simulink model "pfb_fft_vacc_float_fft"  (R2016b)
settings: Solver=VariableStepAuto  StartTime=0.0  StopTime=1024*2^5  RelTol=1e-3
undefined symbols (not defined in the file): fft_stages
libraries: xbsIndex_r4 x17526, casper_library_misc x189, casper_library_delays x15, casper_library_sources x4, xbsReplacements_r4 x3, xps_library x1
(part 30 of 140)

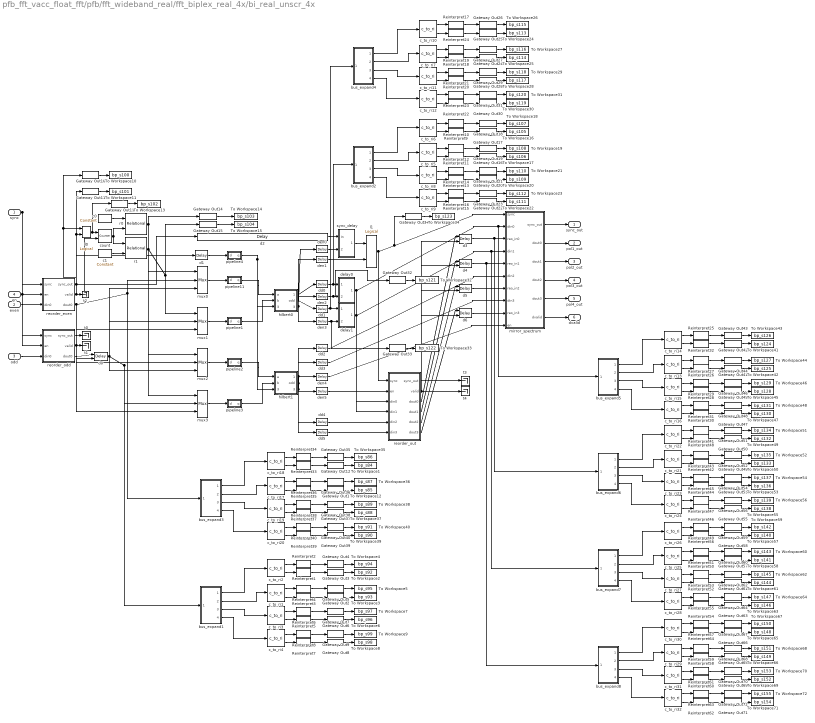
== system pfb_fft_vacc_float_fft/pfb/fft_wideband_real/fft_biplex_real_4x/bi_real_unscr_4x ==
  sync : Inport
  even : Inport  Port=2
  odd : Inport  Port=3
  en : Inport  Port=4
  Gateway Out{1..72} (72 alike) : xbsIndex_r4/Gateway Out  inherit_from_input=off  hdl_port=on  rows=1  columns=1  UseAsDAC=off  DACChannel='1'  interface=None  automatic_assignment=on  address_offset=hex2dec('00')  intf_name=''  intf_description=''  timing_constraint=None  locs_specified=off  LOCs={}  io_standard={}  block_type=gatewayout
  Reinterpret{1..64} (64 alike) : xbsIndex_r4/Reinterpret  force_arith_type=on  arith_type=Floating-point  force_bin_pt=on  bin_pt=24  block_type=reinterpret
  To Workspace1 : ToWorkspace  VariableName=bp_s84  SaveFormat=Timeseries  SampleTime=-1
  To Workspace10 : ToWorkspace  VariableName=bp_s100  SaveFormat=Timeseries  SampleTime=-1
  To Workspace11 : ToWorkspace  VariableName=bp_s101  SaveFormat=Timeseries  SampleTime=-1
  To Workspace12 : ToWorkspace  VariableName=bp_s85  SaveFormat=Timeseries  SampleTime=-1
  To Workspace13 : ToWorkspace  VariableName=bp_s102  SaveFormat=Timeseries  SampleTime=-1
  To Workspace14 : ToWorkspace  VariableName=bp_s103  SaveFormat=Timeseries  SampleTime=-1
  To Workspace15 : ToWorkspace  VariableName=bp_s104  SaveFormat=Timeseries  SampleTime=-1
  To Workspace16 : ToWorkspace  VariableName=bp_s105  SaveFormat=Timeseries  SampleTime=-1
  To Workspace17 : ToWorkspace  VariableName=bp_s106  SaveFormat=Timeseries  SampleTime=-1
  To Workspace18 : ToWorkspace  VariableName=bp_s107  SaveFormat=Timeseries  SampleTime=-1
  To Workspace19 : ToWorkspace  VariableName=bp_s108  SaveFormat=Timeseries  SampleTime=-1
  To Workspace2 : ToWorkspace  VariableName=bp_s92  SaveFormat=Timeseries  SampleTime=-1
  To Workspace20 : ToWorkspace  VariableName=bp_s109  SaveFormat=Timeseries  SampleTime=-1
  To Workspace21 : ToWorkspace  VariableName=bp_s110  SaveFormat=Timeseries  SampleTime=-1
  To Workspace22 : ToWorkspace  VariableName=bp_s111  SaveFormat=Timeseries  SampleTime=-1
  To Workspace23 : ToWorkspace  VariableName=bp_s112  SaveFormat=Timeseries  SampleTime=-1
  To Workspace24 : ToWorkspace  VariableName=bp_s113  SaveFormat=Timeseries  SampleTime=-1
  To Workspace25 : ToWorkspace  VariableName=bp_s114  SaveFormat=Timeseries  SampleTime=-1
  To Workspace26 : ToWorkspace  VariableName=bp_s115  SaveFormat=Timeseries  SampleTime=-1
  To Workspace27 : ToWorkspace  VariableName=bp_s116  SaveFormat=Timeseries  SampleTime=-1
  To Workspace28 : ToWorkspace  VariableName=bp_s117  SaveFormat=Timeseries  SampleTime=-1
  To Workspace29 : ToWorkspace  VariableName=bp_s118  SaveFormat=Timeseries  SampleTime=-1
  To Workspace3 : ToWorkspace  VariableName=bp_s93  SaveFormat=Timeseries  SampleTime=-1
  To Workspace30 : ToWorkspace  VariableName=bp_s119  SaveFormat=Timeseries  SampleTime=-1
  To Workspace31 : ToWorkspace  VariableName=bp_s120  SaveFormat=Timeseries  SampleTime=-1
  To Workspace32 : ToWorkspace  VariableName=bp_s121  SaveFormat=Timeseries  SampleTime=-1
  To Workspace33 : ToWorkspace  VariableName=bp_s122  SaveFormat=Timeseries  SampleTime=-1
  To Workspace34 : ToWorkspace  VariableName=bp_s123  SaveFormat=Timeseries  SampleTime=-1
  To Workspace35 : ToWorkspace  VariableName=bp_s86  SaveFormat=Timeseries  SampleTime=-1
  To Workspace36 : ToWorkspace  VariableName=bp_s87  SaveFormat=Timeseries  SampleTime=-1
  To Workspace37 : ToWorkspace  VariableName=bp_s88  SaveFormat=Timeseries  SampleTime=-1
  To Workspace38 : ToWorkspace  VariableName=bp_s89  SaveFormat=Timeseries  SampleTime=-1
  To Workspace39 : ToWorkspace  VariableName=bp_s90  SaveFormat=Timeseries  SampleTime=-1
  To Workspace4 : ToWorkspace  VariableName=bp_s94  SaveFormat=Timeseries  SampleTime=-1
  To Workspace40 : ToWorkspace  VariableName=bp_s91  SaveFormat=Timeseries  SampleTime=-1
  To Workspace41 : ToWorkspace  VariableName=bp_s124  SaveFormat=Timeseries  SampleTime=-1
  To Workspace42 : ToWorkspace  VariableName=bp_s125  SaveFormat=Timeseries  SampleTime=-1
  To Workspace43 : ToWorkspace  VariableName=bp_s126  SaveFormat=Timeseries  SampleTime=-1
  To Workspace44 : ToWorkspace  VariableName=bp_s127  SaveFormat=Timeseries  SampleTime=-1
  To Workspace45 : ToWorkspace  VariableName=bp_s128  SaveFormat=Timeseries  SampleTime=-1
  To Workspace46 : ToWorkspace  VariableName=bp_s129  SaveFormat=Timeseries  SampleTime=-1
  To Workspace47 : ToWorkspace  VariableName=bp_s130  SaveFormat=Timeseries  SampleTime=-1
  To Workspace48 : ToWorkspace  VariableName=bp_s131  SaveFormat=Timeseries  SampleTime=-1
  To Workspace49 : ToWorkspace  VariableName=bp_s132  SaveFormat=Timeseries  SampleTime=-1
  To Workspace5 : ToWorkspace  VariableName=bp_s95  SaveFormat=Timeseries  SampleTime=-1
  To Workspace50 : ToWorkspace  VariableName=bp_s133  SaveFormat=Timeseries  SampleTime=-1
  To Workspace51 : ToWorkspace  VariableName=bp_s134  SaveFormat=Timeseries  SampleTime=-1
  To Workspace52 : ToWorkspace  VariableName=bp_s135  SaveFormat=Timeseries  SampleTime=-1
  To Workspace53 : ToWorkspace  VariableName=bp_s136  SaveFormat=Timeseries  SampleTime=-1
  To Workspace54 : ToWorkspace  VariableName=bp_s137  SaveFormat=Timeseries  SampleTime=-1
  To Workspace55 : ToWorkspace  VariableName=bp_s138  SaveFormat=Timeseries  SampleTime=-1
  To Workspace56 : ToWorkspace  VariableName=bp_s139  SaveFormat=Timeseries  SampleTime=-1
  To Workspace57 : ToWorkspace  VariableName=bp_s140  SaveFormat=Timeseries  SampleTime=-1
  To Workspace58 : ToWorkspace  VariableName=bp_s141  SaveFormat=Timeseries  SampleTime=-1
  To Workspace59 : ToWorkspace  VariableName=bp_s142  SaveFormat=Timeseries  SampleTime=-1
  To Workspace6 : ToWorkspace  VariableName=bp_s96  SaveFormat=Timeseries  SampleTime=-1
  To Workspace60 : ToWorkspace  VariableName=bp_s143  SaveFormat=Timeseries  SampleTime=-1
  To Workspace61 : ToWorkspace  VariableName=bp_s144  SaveFormat=Timeseries  SampleTime=-1
  To Workspace62 : ToWorkspace  VariableName=bp_s145  SaveFormat=Timeseries  SampleTime=-1
  To Workspace63 : ToWorkspace  VariableName=bp_s146  SaveFormat=Timeseries  SampleTime=-1
  To Workspace64 : ToWorkspace  VariableName=bp_s147  SaveFormat=Timeseries  SampleTime=-1
  To Workspace65 : ToWorkspace  VariableName=bp_s148  SaveFormat=Timeseries  SampleTime=-1
  To Workspace66 : ToWorkspace  VariableName=bp_s149  SaveFormat=Timeseries  SampleTime=-1
  To Workspace67 : ToWorkspace  VariableName=bp_s150  SaveFormat=Timeseries  SampleTime=-1
  To Workspace68 : ToWorkspace  VariableName=bp_s151  SaveFormat=Timeseries  SampleTime=-1
  To Workspace69 : ToWorkspace  VariableName=bp_s152  SaveFormat=Timeseries  SampleTime=-1
  To Workspace7 : ToWorkspace  VariableName=bp_s97  SaveFormat=Timeseries  SampleTime=-1
  To Workspace70 : ToWorkspace  VariableName=bp_s153  SaveFormat=Timeseries  SampleTime=-1
  To Workspace71 : ToWorkspace  VariableName=bp_s154  SaveFormat=Timeseries  SampleTime=-1
  To Workspace72 : ToWorkspace  VariableName=bp_s155  SaveFormat=Timeseries  SampleTime=-1
  To Workspace8 : ToWorkspace  VariableName=bp_s98  SaveFormat=Timeseries  SampleTime=-1
  To Workspace9 : ToWorkspace  VariableName=bp_s99  SaveFormat=Timeseries  SampleTime=-1
  bus_expand1 : Subsystem  AncestorBlock=casper_library_flow_control/bus_expand  mask(bus_expand){mode=divisions of equal size, outputNum=4, outputWidth=[64 64 64 64], outputBinaryPt=[0 0  0 0], outputArithmeticType=[0 0  0 0], show_format=off, outputToWorkspace=off, variablePrefix=out, outputToModelAsWell=on}
      mask prompts: outputNum "Number of outputs:"; outputWidth "Output width:"; outputBinaryPt "Output binary point position:"; outputArithmeticType "Output arithmetic type (ufix=0, fix=1, bool=2):"; show_format "Print format string?"; outputToWorkspace "Output to workspace?"; variablePrefix "Variable name prefix:"; outputToModelAsWell "Output to model as well?"
      mask help:
      | Expands a 'bus' made using a bus_create or Xilinx
      | concat block.
      | The input will be divided into the specified number
      | of outputs with the specified arithmetic types.
      | Divisions are made from most significant bit.
      mask init: same as pfb_fft_vacc_float_fft/bus_expand1
      settings: AttributesFormatString=4 outputs
      mask TypeOptions mode: divisions of equal size | divisions of arbitrary size
      mask Callback mode: bus_expand_callback();
      mask Callback outputWidth: bus_expand_callback();
      mask Callback outputToWorkspace: bus_expand_callback();
  bus_expand2 : Subsystem  AncestorBlock=casper_library_flow_control/bus_expand  mask(bus_expand){mode=divisions of equal size, outputNum=4, outputWidth=[64 64 64 64], outputBinaryPt=[0 0  0 0], outputArithmeticType=[0 0  0 0], show_format=off, outputToWorkspace=off, variablePrefix=out, outputToModelAsWell=on}
      mask prompts: outputNum "Number of outputs:"; outputWidth "Output width:"; outputBinaryPt "Output binary point position:"; outputArithmeticType "Output arithmetic type (ufix=0, fix=1, bool=2):"; show_format "Print format string?"; outputToWorkspace "Output to workspace?"; variablePrefix "Variable name prefix:"; outputToModelAsWell "Output to model as well?"
      mask help:
      | Expands a 'bus' made using a bus_create or Xilinx
      | concat block.
      | The input will be divided into the specified number
      | of outputs with the specified arithmetic types.
      | Divisions are made from most significant bit.
      mask init: same as pfb_fft_vacc_float_fft/bus_expand1
      settings: AttributesFormatString=4 outputs
      mask TypeOptions mode: divisions of equal size | divisions of arbitrary size
      mask Callback mode: bus_expand_callback();
      mask Callback outputWidth: bus_expand_callback();
      mask Callback outputToWorkspace: bus_expand_callback();
  bus_expand3 : Subsystem  AncestorBlock=casper_library_flow_control/bus_expand  mask(bus_expand){mode=divisions of equal size, outputNum=4, outputWidth=[64 64 64 64], outputBinaryPt=[0 0  0 0], outputArithmeticType=[0 0  0 0], show_format=off, outputToWorkspace=off, variablePrefix=out, outputToModelAsWell=on}
      mask prompts: outputNum "Number of outputs:"; outputWidth "Output width:"; outputBinaryPt "Output binary point position:"; outputArithmeticType "Output arithmetic type (ufix=0, fix=1, bool=2):"; show_format "Print format string?"; outputToWorkspace "Output to workspace?"; variablePrefix "Variable name prefix:"; outputToModelAsWell "Output to model as well?"
      mask help:
      | Expands a 'bus' made using a bus_create or Xilinx
      | concat block.
      | The input will be divided into the specified number
      | of outputs with the specified arithmetic types.
      | Divisions are made from most significant bit.
      mask init: same as pfb_fft_vacc_float_fft/bus_expand1
      settings: AttributesFormatString=4 outputs
      mask TypeOptions mode: divisions of equal size | divisions of arbitrary size
      mask Callback mode: bus_expand_callback();
      mask Callback outputWidth: bus_expand_callback();
      mask Callback outputToWorkspace: bus_expand_callback();
  bus_expand4 : Subsystem  AncestorBlock=casper_library_flow_control/bus_expand  mask(bus_expand){mode=divisions of equal size, outputNum=4, outputWidth=[64 64 64 64], outputBinaryPt=[0 0  0 0], outputArithmeticType=[0 0  0 0], show_format=off, outputToWorkspace=off, variablePrefix=out, outputToModelAsWell=on}
      mask prompts: outputNum "Number of outputs:"; outputWidth "Output width:"; outputBinaryPt "Output binary point position:"; outputArithmeticType "Output arithmetic type (ufix=0, fix=1, bool=2):"; show_format "Print format string?"; outputToWorkspace "Output to workspace?"; variablePrefix "Variable name prefix:"; outputToModelAsWell "Output to model as well?"
      mask help:
      | Expands a 'bus' made using a bus_create or Xilinx
      | concat block.
      | The input will be divided into the specified number
      | of outputs with the specified arithmetic types.
      | Divisions are made from most significant bit.
      mask init: same as pfb_fft_vacc_float_fft/bus_expand1
      settings: AttributesFormatString=4 outputs
      mask TypeOptions mode: divisions of equal size | divisions of arbitrary size
      mask Callback mode: bus_expand_callback();
      mask Callback outputWidth: bus_expand_callback();
      mask Callback outputToWorkspace: bus_expand_callback();
  bus_expand5 : Subsystem  AncestorBlock=casper_library_flow_control/bus_expand  mask(bus_expand){mode=divisions of equal size, outputNum=4, outputWidth=[64 64 64 64], outputBinaryPt=[0 0  0 0], outputArithmeticType=[0 0  0 0], show_format=off, outputToWorkspace=off, variablePrefix=out, outputToModelAsWell=on}
      mask prompts: outputNum "Number of outputs:"; outputWidth "Output width:"; outputBinaryPt "Output binary point position:"; outputArithmeticType "Output arithmetic type (ufix=0, fix=1, bool=2):"; show_format "Print format string?"; outputToWorkspace "Output to workspace?"; variablePrefix "Variable name prefix:"; outputToModelAsWell "Output to model as well?"
      mask help:
      | Expands a 'bus' made using a bus_create or Xilinx
      | concat block.
      | The input will be divided into the specified number
      | of outputs with the specified arithmetic types.
      | Divisions are made from most significant bit.
      mask init: same as pfb_fft_vacc_float_fft/bus_expand1
      settings: AttributesFormatString=4 outputs
      mask TypeOptions mode: divisions of equal size | divisions of arbitrary size
      mask Callback mode: bus_expand_callback();
      mask Callback outputWidth: bus_expand_callback();
      mask Callback outputToWorkspace: bus_expand_callback();
  bus_expand6 : Subsystem  AncestorBlock=casper_library_flow_control/bus_expand  mask(bus_expand){mode=divisions of equal size, outputNum=4, outputWidth=[64 64 64 64], outputBinaryPt=[0 0  0 0], outputArithmeticType=[0 0  0 0], show_format=off, outputToWorkspace=off, variablePrefix=out, outputToModelAsWell=on}
      mask prompts: outputNum "Number of outputs:"; outputWidth "Output width:"; outputBinaryPt "Output binary point position:"; outputArithmeticType "Output arithmetic type (ufix=0, fix=1, bool=2):"; show_format "Print format string?"; outputToWorkspace "Output to workspace?"; variablePrefix "Variable name prefix:"; outputToModelAsWell "Output to model as well?"
      mask help:
      | Expands a 'bus' made using a bus_create or Xilinx
      | concat block.
      | The input will be divided into the specified number
      | of outputs with the specified arithmetic types.
      | Divisions are made from most significant bit.
      mask init: same as pfb_fft_vacc_float_fft/bus_expand1
      settings: AttributesFormatString=4 outputs
      mask TypeOptions mode: divisions of equal size | divisions of arbitrary size
      mask Callback mode: bus_expand_callback();
      mask Callback outputWidth: bus_expand_callback();
      mask Callback outputToWorkspace: bus_expand_callback();
  bus_expand7 : Subsystem  AncestorBlock=casper_library_flow_control/bus_expand  mask(bus_expand){mode=divisions of equal size, outputNum=4, outputWidth=[64 64 64 64], outputBinaryPt=[0 0  0 0], outputArithmeticType=[0 0  0 0], show_format=off, outputToWorkspace=off, variablePrefix=out, outputToModelAsWell=on}
      mask prompts: outputNum "Number of outputs:"; outputWidth "Output width:"; outputBinaryPt "Output binary point position:"; outputArithmeticType "Output arithmetic type (ufix=0, fix=1, bool=2):"; show_format "Print format string?"; outputToWorkspace "Output to workspace?"; variablePrefix "Variable name prefix:"; outputToModelAsWell "Output to model as well?"
      mask help:
      | Expands a 'bus' made using a bus_create or Xilinx
      | concat block.
      | The input will be divided into the specified number
      | of outputs with the specified arithmetic types.
      | Divisions are made from most significant bit.
      mask init: same as pfb_fft_vacc_float_fft/bus_expand1
      settings: AttributesFormatString=4 outputs
      mask TypeOptions mode: divisions of equal size | divisions of arbitrary size
      mask Callback mode: bus_expand_callback();
      mask Callback outputWidth: bus_expand_callback();
      mask Callback outputToWorkspace: bus_expand_callback();
  bus_expand8 : Subsystem  AncestorBlock=casper_library_flow_control/bus_expand  mask(bus_expand){mode=divisions of equal size, outputNum=4, outputWidth=[64 64 64 64], outputBinaryPt=[0 0  0 0], outputArithmeticType=[0 0  0 0], show_format=off, outputToWorkspace=off, variablePrefix=out, outputToModelAsWell=on}
      mask prompts: outputNum "Number of outputs:"; outputWidth "Output width:"; outputBinaryPt "Output binary point position:"; outputArithmeticType "Output arithmetic type (ufix=0, fix=1, bool=2):"; show_format "Print format string?"; outputToWorkspace "Output to workspace?"; variablePrefix "Variable name prefix:"; outputToModelAsWell "Output to model as well?"
      mask help:
      | Expands a 'bus' made using a bus_create or Xilinx
      | concat block.
      | The input will be divided into the specified number
      | of outputs with the specified arithmetic types.
      | Divisions are made from most significant bit.
      mask init: same as pfb_fft_vacc_float_fft/bus_expand1
      settings: AttributesFormatString=4 outputs
      mask TypeOptions mode: divisions of equal size | divisions of arbitrary size
      mask Callback mode: bus_expand_callback();
      mask Callback outputWidth: bus_expand_callback();
      mask Callback outputToWorkspace: bus_expand_callback();
  c0 : xbsIndex_r4/Constant  const=2^(FFTSize-1)  gui_display_data_type=Fixed-point  arith_type=Unsigned  n_bits=FFTSize  bin_pt=0  preci_type=Single  exp_width=8  frac_width=24  explicit_period=on  period=1  equ=P=C  opselect=C  inp2=PCIN>>17  opr=+  inp1=P  carry=CIN  dbl_ovrd=off  block_type=constant
  c1 : xbsIndex_r4/Constant  const=0  gui_display_data_type=Fixed-point  arith_type=Unsigned  n_bits=FFTSize  bin_pt=0  preci_type=Single  exp_width=8  frac_width=24  explicit_period=on  period=1  equ=P=C  opselect=C  inp2=PCIN>>17  opr=+  inp1=P  carry=CIN  dbl_ovrd=off  block_type=constant
  c_to_ri{1..32} (32 alike) : casper_library_misc/c_to_ri  n_bits=32  bin_pt=0
      settings: AttributesFormatString=32_0 r/i
  count : xbsIndex_r4/Counter  cnt_type=Free Running  cnt_to=Inf  operation=Up  start_count=0  cnt_by_val=1  arith_type=Unsigned  n_bits=FFTSize  bin_pt=0  load_pin=off  rst=on  en=on  explicit_period=on  period=1  use_behavioral_HDL=off  implementation=Fabric  dbl_ovrd=off  block_type=counter
  d{0,3..6} (5 alike) : xbsIndex_r4/Delay  rst=off  infoeditControl=Selection of Reset will increase slice count due to use of real FFs and instead of SRLs  en=on  latency=1  reg_retiming=on  dbl_ovrd=off  block_type=delay
      settings: infoedit=Hardware notes: A delay line is a chain, each link of which is an SRL16 or SRL32 followed by a flip-flop.
  d1 : xbsIndex_r4/Delay  rst=off  infoeditControl=Selection of Reset will increase slice count due to use of real FFs and instead of SRLs  en=off  latency=1  reg_retiming=on  dbl_ovrd=off  block_type=delay
      settings: infoedit=Hardware notes: A delay line is a chain, each link of which is an SRL16 or SRL32 followed by a flip-flop.
  d2 : xbsIndex_r4/Delay  rst=off  infoeditControl=Selection of Reset will increase slice count due to use of real FFs and instead of SRLs  en=off  latency=add_latency + conv_latency + 1+2  reg_retiming=on  dbl_ovrd=off  block_type=delay
      settings: infoedit=Hardware notes: A delay line is a chain, each link of which is an SRL16 or SRL32 followed by a flip-flop.
  dd{0..5} (6 alike) : xbsIndex_r4/Delay  rst=off  infoeditControl=Selection of Reset will increase slice count due to use of real FFs and instead of SRLs  en=off  latency=1  reg_retiming=on  dbl_ovrd=off  block_type=delay
      settings: infoedit=Hardware notes: A delay line is a chain, each link of which is an SRL16 or SRL32 followed by a flip-flop.
  delay0 : Subsystem  AncestorBlock=casper_library_delays/delay_bram  mask(delay_bram){DelayLen=512, bram_latency=4, use_dsp48=off, async=on}
      mask prompts: DelayLen "Delay By:"; bram_latency "BRAM Latency"; use_dsp48 "Use DSP48"; async "asynchronous operation"
      mask help: A delay block that uses BRAM for its storage.
      mask init:
      | delay_bram_init(gcb, ...
      |     'DelayLen', DelayLen, ...
      |     'bram_latency', bram_latency, ...
      |     'use_dsp48', use_dsp48, ...
      |     'async', async);
      mask Help: eval('xlWeb(''http://casper.berkeley.edu/wiki/Delay_bram'')')
  delay1 : Subsystem  AncestorBlock=casper_library_delays/delay_bram  mask(delay_bram){DelayLen=512, bram_latency=4, use_dsp48=off, async=on}
      mask prompts: DelayLen "Delay By:"; bram_latency "BRAM Latency"; use_dsp48 "Use DSP48"; async "asynchronous operation"
      mask help: A delay block that uses BRAM for its storage.
      mask init:
      | delay_bram_init(gcb, ...
      |     'DelayLen', DelayLen, ...
      |     'bram_latency', bram_latency, ...
      |     'use_dsp48', use_dsp48, ...
      |     'async', async);
      mask Help: eval('xlWeb(''http://casper.berkeley.edu/wiki/Delay_bram'')')
  den{0..5} (6 alike) : xbsIndex_r4/Delay  rst=off  infoeditControl=Selection of Reset will increase slice count due to use of real FFs and instead of SRLs  en=off  latency=1  reg_retiming=off  dbl_ovrd=off  block_type=delay
      settings: infoedit=Hardware notes: A delay line is a chain, each link of which is an SRL16 or SRL32 followed by a flip-flop.
  hilbert0 : Subsystem  AncestorBlock=casper_library_ffts_internal/hilbert  mask(hilbert){n_inputs=4, BitWidth=32, bin_pt_in=0, add_latency=2, conv_latency=conv_latency, misc=on}
      mask prompts: n_inputs "Number simultaneous inputs"; BitWidth "Bit Width"; bin_pt_in "Binary point in"; add_latency "Add Latency"; conv_latency "Convert Latency"; misc "Miscellaneous input"
      mask help:
      | FFT internal block.
      |
      | Computes (a+b*)/2 and (a-b*)/2i.
      |
      | If a = w + ix and b = y + iz, then:
      | a+b = (w+y)/2 + i(x-z)/2
      | a-b = (x+z)/2 + i(y-w)/2
      mask init:
      | hilbert_init(gcb, ...
      |     'n_inputs', n_inputs, ...
      |     'BitWidth', BitWidth, ...
      |     'bin_pt_in', bin_pt_in, ...
      |     'add_latency', add_latency, ...
      |     'conv_latency', conv_latency, ...
      |     'misc', misc);
  hilbert1 : Subsystem  AncestorBlock=casper_library_ffts_internal/hilbert  mask(hilbert){n_inputs=4, BitWidth=32, bin_pt_in=0, add_latency=2, conv_latency=conv_latency, misc=on}
      mask prompts: n_inputs "Number simultaneous inputs"; BitWidth "Bit Width"; bin_pt_in "Binary point in"; add_latency "Add Latency"; conv_latency "Convert Latency"; misc "Miscellaneous input"
      mask help:
      | FFT internal block.
      |
      | Computes (a+b*)/2 and (a-b*)/2i.
      |
      | If a = w + ix and b = y + iz, then:
      | a+b = (w+y)/2 + i(x-z)/2
      | a-b = (x+z)/2 + i(y-w)/2
      mask init: same as pfb_fft_vacc_float_fft/pfb/fft_wideband_real/fft_biplex_real_4x/bi_real_unscr_4x/hilbert0
  l0 : xbsIndex_r4/Logical  logical_function=AND  inputs=2  en=off  latency=0  precision=Full  arith_type=Unsigned  n_bits=16  bin_pt=0  align_bp=on  dbl_ovrd=off  block_type=logical
  l1 : xbsIndex_r4/Logical  logical_function=AND  inputs=2  en=off  latency=0  precision=Full  arith_type=Unsigned  n_bits=16  bin_pt=0  align_bp=on  dbl_ovrd=off  block_type=logical
  mirror_spectrum : Subsystem  AncestorBlock=casper_library_ffts_internal/mirror_spectrum  mask(mirror_spectrum){n_inputs=4, FFTSize=10, input_bitwidth=32, bin_pt_in=0, bram_latency=10, negate_latency=0, negate_mode=logic, async=on}
      mask prompts: n_inputs "Number simultaneous inputs (2*?)"; FFTSize "Size of FFT (2^?)"; input_bitwidth "Input Bit Width"; bin_pt_in "Binary point in"; bram_latency "BRAM Latency"; negate_latency "Negate Latency"; negate_mode "Negate mode"; async "Asynchronous operation"
      mask help: FFT internal block.
      mask init:
      | mirror_spectrum_init(gcb, ...
      |     'n_inputs', n_inputs, ...
      |     'FFTSize', FFTSize, ...
      |     'input_bitwidth', input_bitwidth, ...
      |     'bin_pt_in', bin_pt_in, ...
      |     'bram_latency', bram_latency, ...
      |     'negate_latency', negate_latency, ...
      |     'negate_mode', negate_mode, ...
      |     'async', async);
      mask TypeOptions negate_mode: dsp48e | logic
  mux{0..3} (4 alike) : xbsIndex_r4/Mux  inputs=2  en=off  latency=1  precision=Full  arith_type=Unsigned  n_bits=16  bin_pt=14  quantization=Truncate  overflow=Wrap  dbl_ovrd=off  block_type=mux
  pipeline1 : Subsystem  AncestorBlock=casper_library_delays/pipeline  mask(pipeline){latency=1}
      mask help:
      | An explicitly laid-out delay line to avoid mapping to SRL16s
      | for use in pipelining to help achieve timing closure.
      mask init:
      | pipeline_init(gcb,'latency',latency);
      settings: AttributesFormatString=Latency = %<latency>
      mask Display:
      | port_label('input', 1, 'd');
      | port_label('output', 1, 'q');
      | disp(['Z^-', num2str(latency)]);
  pipeline11 : Subsystem  AncestorBlock=casper_library_delays/pipeline  mask(pipeline){latency=1}
      mask help:
      | An explicitly laid-out delay line to avoid mapping to SRL16s
      | for use in pipelining to help achieve timing closure.
      mask init:
      | pipeline_init(gcb,'latency',latency);
      settings: AttributesFormatString=Latency = %<latency>
      mask Display:
      | port_label('input', 1, 'd');
      | port_label('output', 1, 'q');
      | disp(['Z^-', num2str(latency)]);
  pipeline2 : Subsystem  AncestorBlock=casper_library_delays/pipeline  mask(pipeline){latency=1}
      mask help:
      | An explicitly laid-out delay line to avoid mapping to SRL16s
      | for use in pipelining to help achieve timing closure.
      mask init:
      | pipeline_init(gcb,'latency',latency);
      settings: AttributesFormatString=Latency = %<latency>
      mask Display:
      | port_label('input', 1, 'd');
      | port_label('output', 1, 'q');
      | disp(['Z^-', num2str(latency)]);
  pipeline3 : Subsystem  AncestorBlock=casper_library_delays/pipeline  mask(pipeline){latency=1}
      mask help:
      | An explicitly laid-out delay line to avoid mapping to SRL16s
      | for use in pipelining to help achieve timing closure.
      mask init:
      | pipeline_init(gcb,'latency',latency);
      settings: AttributesFormatString=Latency = %<latency>
      mask Display:
      | port_label('input', 1, 'd');
      | port_label('output', 1, 'q');
      | disp(['Z^-', num2str(latency)]);
  pipeline4 : Subsystem  AncestorBlock=casper_library_delays/pipeline  mask(pipeline){latency=1}
      mask help:
      | An explicitly laid-out delay line to avoid mapping to SRL16s
      | for use in pipelining to help achieve timing closure.
      mask init:
      | pipeline_init(gcb,'latency',latency);
      settings: AttributesFormatString=Latency = %<latency>
      mask Display:
      | port_label('input', 1, 'd');
      | port_label('output', 1, 'q');
      | disp(['Z^-', num2str(latency)]);
  r0 : xbsIndex_r4/Relational  mode=a=b  op_type=Bool  en=off  latency=0  dbl_ovrd=off  block_type=relational
  r1 : xbsIndex_r4/Relational  mode=a=b  op_type=Bool  en=off  latency=0  dbl_ovrd=off  block_type=relational
  reorder_even : Subsystem  AncestorBlock=casper_library_reorder/reorder  mask(reorder){map=[0 256 128 384 64 320 192 448 32 288 160 416 96 352 224 480 16 272 144 400 80 336 208 464 48 304 176 432 112 368 240 496 8 264 136 392 72 328 200 456 40 296 168 424 104 360 232 488 24 280 152 408 88 344 216 472 56 312 184 440 120 376 248 504 4 260 132 388 68 324 196 452 36 292 164 420 100 356 228 484 20 276 148 404 84 340 212 468 52 308 180 436 116 372 244 500 12 268 140 396 76 332 204 460 44 300 172 428 108 364 236 492 28 284 156 412 92 348 220 476 60 316 188 444 124 380 252 508 2 258 130 386 66 322 194 450 34 290 162 418 98 354 226 482 18 274 146 402 82 338 210 466 50 306 178 434 114 370 242 498 10 266 138 394 74 330 202 458 42 298 170 426 106 362 234 490 26 282 154 410 90 346 218 474 58 314 186 442 122 378 250 506 6 262 134 390 70 326 198 454 38 294 166 422 102 358 230 486 22 278 150 406 86 342 214 470 54 310 182 438 118 374 246 502 14 270 142 398 78 334 206 462 46 302 174 430 110 366 238 494 30 286 158 414 94 350 222 478 62 318 190 446 126 382 254 510 1 257 129 385 65 321 193 449 33 289 161 417 97 353 225 481 17 273 145 401 81 337 209 465 49 305 177 433 113 369 241 497 9 265 137 393 73 329 201 457 41 297 169 425 105 361 233 489 25 281 153 409 89 345 217 473 57 313 185 441 121 377 249 505 5 261 133 389 69 325 197 453 37 293 165 421 101 357 229 485 21 277 149 405 85 341 213 469 53 309 181 437 117 373 245 501 13 269 141 397 77 333 205 461 45 301 173 429 109 365 237 493 29 285 157 413 93 349 221 477 61 317 189 445 125 381 253 509 3 259 131 387 67 323 195 451 35 291 163 419 99 355 227 483 19 275 147 403 83 339 211 467 51 307 179 435 115 371 243 499 11 267 139 395 75 331 203 459 43 299 171 427 107 363 235 491 27 283 155 411 91 347 219 475 59 315 187 443 123 379 251 507 7 263 135 391 71 327 199 455 39 295 167 423 103 359 231 487 23 279 151 407 87 343 215 471 55 311 183 439 119 375 247 503 15 271 143 399 79 335 207 463 47 303 175 431 111 367 239 495 31 287 159 415 95 351 223 479 63 319 191 447 127 383 255 511], n_bits=256, n_inputs=1, bram_latency=4, map_latency=1, fanout_latency=3, double_buffer=0, bram_map=off, software_controlled=off}
      mask prompts: map "Output Order:"; n_bits "Data width in"; n_inputs "Number of inputs"; bram_latency "BRAM Latency"; map_latency "Map Latency"; fanout_latency "Fanout latency"; double_buffer "Double Buffer"; bram_map "Use BlockRAM for Map"; software_controlled "Use runtime software control"
      mask init: same as pfb_fft_vacc_float_fft/pfb/fft_wideband_real/fft_unscrambler/reorder
      settings: AttributesFormatString=order=2
      mask Help: eval('xlWeb(''http://casper.berkeley.edu/wiki/Reorder'')')
  reorder_odd : Subsystem  AncestorBlock=casper_library_reorder/reorder  mask(reorder){map=[511 255 383 127 447 191 319 63 479 223 351 95 415 159 287 31 495 239 367 111 431 175 303 47 463 207 335 79 399 143 271 15 503 247 375 119 439 183 311 55 471 215 343 87 407 151 279 23 487 231 359 103 423 167 295 39 455 199 327 71 391 135 263 7 507 251 379 123 443 187 315 59 475 219 347 91 411 155 283 27 491 235 363 107 427 171 299 43 459 203 331 75 395 139 267 11 499 243 371 115 435 179 307 51 467 211 339 83 403 147 275 19 483 227 355 99 419 163 291 35 451 195 323 67 387 131 259 3 509 253 381 125 445 189 317 61 477 221 349 93 413 157 285 29 493 237 365 109 429 173 301 45 461 205 333 77 397 141 269 13 501 245 373 117 437 181 309 53 469 213 341 85 405 149 277 21 485 229 357 101 421 165 293 37 453 197 325 69 389 133 261 5 505 249 377 121 441 185 313 57 473 217 345 89 409 153 281 25 489 233 361 105 425 169 297 41 457 201 329 73 393 137 265 9 497 241 369 113 433 177 305 49 465 209 337 81 401 145 273 17 481 225 353 97 417 161 289 33 449 193 321 65 385 129 257 1 510 254 382 126 446 190 318 62 478 222 350 94 414 158 286 30 494 238 366 110 430 174 302 46 462 206 334 78 398 142 270 14 502 246 374 118 438 182 310 54 470 214 342 86 406 150 278 22 486 230 358 102 422 166 294 38 454 198 326 70 390 134 262 6 506 250 378 122 442 186 314 58 474 218 346 90 410 154 282 26 490 234 362 106 426 170 298 42 458 202 330 74 394 138 266 10 498 242 370 114 434 178 306 50 466 210 338 82 402 146 274 18 482 226 354 98 418 162 290 34 450 194 322 66 386 130 258 2 508 252 380 124 444 188 316 60 476 220 348 92 412 156 284 28 492 236 364 108 428 172 300 44 460 204 332 76 396 140 268 12 500 244 372 116 436 180 308 52 468 212 340 84 404 148 276 20 484 228 356 100 420 164 292 36 452 196 324 68 388 132 260 4 504 248 376 120 440 184 312 56 472 216 344 88 408 152 280 24 488 232 360 104 424 168 296 40 456 200 328 72 392 136 264 8 496 240 368 112 432 176 304 48 464 208 336 80 400 144 272 16 480 224 352 96 416 160 288 32 448 192 320 64 384 128 256 0], n_bits=256, n_inputs=1, bram_latency=4, map_latency=1, fanout_latency=3, double_buffer=0, bram_map=off, software_controlled=off}
      mask prompts: map "Output Order:"; n_bits "Data width in"; n_inputs "Number of inputs"; bram_latency "BRAM Latency"; map_latency "Map Latency"; fanout_latency "Fanout latency"; double_buffer "Double Buffer"; bram_map "Use BlockRAM for Map"; software_controlled "Use runtime software control"
      mask init: same as pfb_fft_vacc_float_fft/pfb/fft_wideband_real/fft_unscrambler/reorder
      settings: AttributesFormatString=order=2
      mask Help: eval('xlWeb(''http://casper.berkeley.edu/wiki/Reorder'')')
  reorder_out : Subsystem  AncestorBlock=casper_library_reorder/reorder  mask(reorder){map=[511 510 509 508 507 506 505 504 503 502 501 500 499 498 497 496 495 494 493 492 491 490 489 488 487 486 485 484 483 482 481 480 479 478 477 476 475 474 473 472 471 470 469 468 467 466 465 464 463 462 461 460 459 458 457 456 455 454 453 452 451 450 449 448 447 446 445 444 443 442 441 440 439 438 437 436 435 434 433 432 431 430 429 428 427 426 425 424 423 422 421 420 419 418 417 416 415 414 413 412 411 410 409 408 407 406 405 404 403 402 401 400 399 398 397 396 395 394 393 392 391 390 389 388 387 386 385 384 383 382 381 380 379 378 377 376 375 374 373 372 371 370 369 368 367 366 365 364 363 362 361 360 359 358 357 356 355 354 353 352 351 350 349 348 347 346 345 344 343 342 341 340 339 338 337 336 335 334 333 332 331 330 329 328 327 326 325 324 323 322 321 320 319 318 317 316 315 314 313 312 311 310 309 308 307 306 305 304 303 302 301 300 299 298 297 296 295 294 293 292 291 290 289 288 287 286 285 284 283 282 281 280 279 278 277 276 275 274 273 272 271 270 269 268 267 266 265 264 263 262 261 260 259 258 257 256 255 254 253 252 251 250 249 248 247 246 245 244 243 242 241 240 239 238 237 236 235 234 233 232 231 230 229 228 227 226 225 224 223 222 221 220 219 218 217 216 215 214 213 212 211 210 209 208 207 206 205 204 203 202 201 200 199 198 197 196 195 194 193 192 191 190 189 188 187 186 185 184 183 182 181 180 179 178 177 176 175 174 173 172 171 170 169 168 167 166 165 164 163 162 161 160 159 158 157 156 155 154 153 152 151 150 149 148 147 146 145 144 143 142 141 140 139 138 137 136 135 134 133 132 131 130 129 128 127 126 125 124 123 122 121 120 119 118 117 116 115 114 113 112 111 110 109 108 107 106 105 104 103 102 101 100 99 98 97 96 95 94 93 92 91 90 89 88 87 86 85 84 83 82 81 80 79 78 77 76 75 74 73 72 71 70 69 68 67 66 65 64 63 62 61 60 59 58 57 56 55 54 53 52 51 50 49 48 47 46 45 44 43 42 41 40 39 38 37 36 35 34 33 32 31 30 29 28 27 26 25 24 23 22 21 20 19 18 17 16 15 14 13 12 11 10 9 8 7 6 5 4 3 2 1 0], n_bits=256, n_inputs=4, bram_latency=bram_latency, map_latency=1, fanout_latency=3, double_buffer=0, bram_map=off, software_controlled=off}
      mask prompts: map "Output Order:"; n_bits "Data width in"; n_inputs "Number of inputs"; bram_latency "BRAM Latency"; map_latency "Map Latency"; fanout_latency "Fanout latency"; double_buffer "Double Buffer"; bram_map "Use BlockRAM for Map"; software_controlled "Use runtime software control"
      mask init: same as pfb_fft_vacc_float_fft/pfb/fft_wideband_real/fft_unscrambler/reorder
      settings: AttributesFormatString=order=2
      mask Help: eval('xlWeb(''http://casper.berkeley.edu/wiki/Reorder'')')
  sync_delay : Subsystem  AncestorBlock=casper_library_delays/sync_delay_en  mask(sync_delay_en){DelayLen=512}
      mask prompts: DelayLen "Delay Length:"
      mask help: Delay an infrequent boolean pulse by the specified number of enabled clocks.
      mask init:
      | BitWidth = max(2, ceil(log2(DelayLen+1)));
      | UseCounter = 1;
      | if DelayLen == 0,
      | 	UseCounter = 0;
      | end
      mask Help: eval('xlWeb(''http://casper.berkeley.edu/wiki/Sync_delay_en'')')
      mask Display:
      | fprintf('Z^-%d', DelayLen);
      | port_label('input',2,'en');
  t{0..4} (5 alike) : Terminator
  sync_out : Outport
  pol1_out : Outport  Port=2
  pol2_out : Outport  Port=3
  pol3_out : Outport  Port=4
  pol4_out : Outport  Port=5
  dvalid : Outport  Port=6
  wires:
  even/1 -> reorder_even/3(din0)
  sync/1 -> reorder_even/1(sync), reorder_odd/1(sync)
  odd/1 -> reorder_odd/3(din0)
  reorder_odd/1(sync_out) -> t0/1
  reorder_odd/2(valid) -> t1/1, d0/2
  reorder_even/2(valid) -> t2/1, Gateway Out11/1, l0/2, count/2, d1/1
  en/1 -> reorder_even/2(en), reorder_odd/2(en)
  reorder_even/1(sync_out) -> l0/1, Gateway Out10/1, d2/1
  c0/1 -> r0/1
  count/1 -> r0/2, r1/1
  c1/1 -> r1/2
  reorder_odd/3(dout0) -> d0/1
  l0/1 -> Gateway Out13/1, count/1
  r0/1 -> Gateway Out14/1, mux0/1, mux3/1
  r1/1 -> Gateway Out15/1, mux1/1, mux2/1
  reorder_even/3(dout0) -> bus_expand3/1(bus_in), mux0/2, mux1/3, mux2/2, mux3/3
  d2/1 -> sync_delay/1(In)
  mux0/1 -> pipeline11/1(d)
  mux1/1 -> pipeline1/1(d)
  mux2/1 -> pipeline2/1(d)
  mux3/1 -> pipeline3/1(d)
  sync_delay/1(Out) -> l1/1
  d1/1 -> pipeline4/1(d)
  hilbert0/3(misco) -> den0/1, den1/1, den2/1, den3/1
  den0/1 -> sync_delay/2(en)
  den1/1 -> l1/2
  hilbert1/3(misco) -> den4/1, den5/1
  hilbert0/1(even) -> dd0/1
  hilbert0/2(odd) -> dd1/1
  hilbert1/1(even) -> dd2/1, dd4/1
  hilbert1/2(odd) -> dd3/1, dd5/1
  dd0/1 -> bus_expand2/1(bus_in), delay0/1(din)
  dd1/1 -> bus_expand4/1(bus_in), delay1/1(din)
  den2/1 -> Gateway Out32/1, delay0/2(en)
  den3/1 -> Gateway Out33/1, delay1/2(en)
  delay0/1(dout) -> bus_expand5/1(bus_in), mirror_spectrum/2(din0), reorder_out/3(din0)
  delay1/1(dout) -> bus_expand7/1(bus_in), mirror_spectrum/4(din1), reorder_out/4(din1)
  dd2/1 -> mirror_spectrum/6(din2)
  dd3/1 -> mirror_spectrum/8(din3)
  mirror_spectrum/1(sync_out) -> sync_out/1
  mirror_spectrum/2(dout0) -> pol1_out/1
  mirror_spectrum/3(dout1) -> pol2_out/1
  mirror_spectrum/4(dout2) -> pol3_out/1
  mirror_spectrum/5(dout3) -> pol4_out/1
  l1/1 -> Gateway Out34/1, mirror_spectrum/1(sync), reorder_out/1(sync)
  den4/1 -> mirror_spectrum/10(en)
  mirror_spectrum/6(dvalid) -> dvalid/1
  dd4/1 -> reorder_out/5(din2)
  dd5/1 -> reorder_out/6(din3)
  den5/1 -> reorder_out/2(en)
  reorder_out/1(sync_out) -> t3/1
  reorder_out/2(valid) -> t4/1, d3/2, d4/2, d5/2, d6/2
  reorder_out/3(dout0) -> d3/1
  d3/1 -> bus_expand6/1(bus_in), mirror_spectrum/3(reo_in0)
  reorder_out/4(dout1) -> d4/1
  d4/1 -> bus_expand8/1(bus_in), mirror_spectrum/5(reo_in1)
  reorder_out/5(dout2) -> d5/1
  d5/1 -> mirror_spectrum/7(reo_in2)
  reorder_out/6(dout3) -> d6/1
  d6/1 -> mirror_spectrum/9(reo_in3)
  Gateway Out12/1 -> To Workspace1/1
  Reinterpret33/1 -> Gateway Out12/1
  Gateway Out35/1 -> To Workspace35/1
  Reinterpret34/1 -> Gateway Out35/1
  c_to_ri18/2 -> Reinterpret33/1
  c_to_ri18/1 -> Reinterpret34/1
  bus_expand3/1(msb_out4) -> c_to_ri18/1
  Gateway Out1/1 -> To Workspace12/1
  Reinterpret35/1 -> Gateway Out1/1
  Gateway Out36/1 -> To Workspace36/1
  Reinterpret36/1 -> Gateway Out36/1
  c_to_ri17/2 -> Reinterpret35/1
  c_to_ri17/1 -> Reinterpret36/1
  Gateway Out37/1 -> To Workspace37/1
  Reinterpret37/1 -> Gateway Out37/1
  Gateway Out38/1 -> To Workspace38/1
  Reinterpret38/1 -> Gateway Out38/1
  c_to_ri19/2 -> Reinterpret37/1
  c_to_ri19/1 -> Reinterpret38/1
  bus_expand3/2(out3) -> c_to_ri17/1
  bus_expand3/3(out2) -> c_to_ri19/1
  Gateway Out39/1 -> To Workspace39/1
  Reinterpret39/1 -> Gateway Out39/1
  Gateway Out40/1 -> To Workspace40/1
  Reinterpret40/1 -> Gateway Out40/1
  c_to_ri20/2 -> Reinterpret39/1
  c_to_ri20/1 -> Reinterpret40/1
  bus_expand3/4(lsb_out1) -> c_to_ri20/1
  Gateway Out3/1 -> To Workspace2/1
  Reinterpret1/1 -> Gateway Out3/1
  Gateway Out4/1 -> To Workspace4/1
  Reinterpret2/1 -> Gateway Out4/1
  c_to_ri2/2 -> Reinterpret1/1
  c_to_ri2/1 -> Reinterpret2/1
  bus_expand1/1(msb_out4) -> c_to_ri2/1
  Gateway Out2/1 -> To Workspace3/1
  Reinterpret3/1 -> Gateway Out2/1
  Gateway Out5/1 -> To Workspace5/1
  Reinterpret4/1 -> Gateway Out5/1
  c_to_ri1/2 -> Reinterpret3/1
  c_to_ri1/1 -> Reinterpret4/1
  Gateway Out6/1 -> To Workspace6/1
  Reinterpret5/1 -> Gateway Out6/1
  Gateway Out7/1 -> To Workspace7/1
  Reinterpret6/1 -> Gateway Out7/1
  c_to_ri3/2 -> Reinterpret5/1
  c_to_ri3/1 -> Reinterpret6/1
  bus_expand1/2(out3) -> c_to_ri1/1
  bus_expand1/3(out2) -> c_to_ri3/1
  Gateway Out8/1 -> To Workspace8/1
  Reinterpret7/1 -> Gateway Out8/1
  Gateway Out9/1 -> To Workspace9/1
  Reinterpret8/1 -> Gateway Out9/1
  c_to_ri4/2 -> Reinterpret7/1
  c_to_ri4/1 -> Reinterpret8/1
  bus_expand1/4(lsb_out1) -> c_to_ri4/1
  d0/1 -> mux0/3, mux1/2, mux2/3, bus_expand1/1(bus_in), mux3/2
  Gateway Out10/1 -> To Workspace10/1
  Gateway Out11/1 -> To Workspace11/1
  Gateway Out13/1 -> To Workspace13/1
  Gateway Out14/1 -> To Workspace14/1
  Gateway Out15/1 -> To Workspace15/1
  Gateway Out17/1 -> To Workspace16/1
  Reinterpret9/1 -> Gateway Out17/1
  Gateway Out18/1 -> To Workspace18/1
  Reinterpret10/1 -> Gateway Out18/1
  c_to_ri6/2 -> Reinterpret9/1
  c_to_ri6/1 -> Reinterpret10/1
  bus_expand2/1(msb_out4) -> c_to_ri6/1
  Gateway Out16/1 -> To Workspace17/1
  Reinterpret11/1 -> Gateway Out16/1
  Gateway Out19/1 -> To Workspace19/1
  Reinterpret12/1 -> Gateway Out19/1
  c_to_ri5/2 -> Reinterpret11/1
  c_to_ri5/1 -> Reinterpret12/1
  Gateway Out20/1 -> To Workspace20/1
  Reinterpret13/1 -> Gateway Out20/1
  Gateway Out21/1 -> To Workspace21/1
  Reinterpret14/1 -> Gateway Out21/1
  c_to_ri8/2 -> Reinterpret13/1
  c_to_ri8/1 -> Reinterpret14/1
  bus_expand2/2(out3) -> c_to_ri5/1
  bus_expand2/3(out2) -> c_to_ri8/1
  Gateway Out22/1 -> To Workspace22/1
  Reinterpret15/1 -> Gateway Out22/1
  Gateway Out23/1 -> To Workspace23/1
  Reinterpret16/1 -> Gateway Out23/1
  c_to_ri9/2 -> Reinterpret15/1
  c_to_ri9/1 -> Reinterpret16/1
  bus_expand2/4(lsb_out1) -> c_to_ri9/1
  Gateway Out25/1 -> To Workspace24/1
  Reinterpret24/1 -> Gateway Out25/1
  Gateway Out26/1 -> To Workspace26/1
  Reinterpret17/1 -> Gateway Out26/1
  c_to_ri10/2 -> Reinterpret24/1
  c_to_ri10/1 -> Reinterpret17/1
  bus_expand4/1(msb_out4) -> c_to_ri10/1
  Gateway Out24/1 -> To Workspace25/1
  Reinterpret18/1 -> Gateway Out24/1
  Gateway Out27/1 -> To Workspace27/1
  Reinterpret19/1 -> Gateway Out27/1
  c_to_ri7/2 -> Reinterpret18/1
  c_to_ri7/1 -> Reinterpret19/1
  Gateway Out28/1 -> To Workspace28/1
  Reinterpret20/1 -> Gateway Out28/1
  Gateway Out29/1 -> To Workspace29/1
  Reinterpret21/1 -> Gateway Out29/1
  c_to_ri11/2 -> Reinterpret20/1
  c_to_ri11/1 -> Reinterpret21/1
  bus_expand4/2(out3) -> c_to_ri7/1
  bus_expand4/3(out2) -> c_to_ri11/1
  Gateway Out30/1 -> To Workspace30/1
  Reinterpret22/1 -> Gateway Out30/1
  Gateway Out31/1 -> To Workspace31/1
  Reinterpret23/1 -> Gateway Out31/1
  c_to_ri12/2 -> Reinterpret22/1
  c_to_ri12/1 -> Reinterpret23/1
  bus_expand4/4(lsb_out1) -> c_to_ri12/1
  Gateway Out32/1 -> To Workspace32/1
  Gateway Out33/1 -> To Workspace33/1
  Gateway Out34/1 -> To Workspace34/1
  Gateway Out42/1 -> To Workspace41/1
  Reinterpret32/1 -> Gateway Out42/1
  Gateway Out43/1 -> To Workspace43/1
  Reinterpret25/1 -> Gateway Out43/1
  c_to_ri14/2 -> Reinterpret32/1
  c_to_ri14/1 -> Reinterpret25/1
  bus_expand5/1(msb_out4) -> c_to_ri14/1
  Gateway Out41/1 -> To Workspace42/1
  Reinterpret26/1 -> Gateway Out41/1
  Gateway Out44/1 -> To Workspace44/1
  Reinterpret27/1 -> Gateway Out44/1
  c_to_ri13/2 -> Reinterpret26/1
  c_to_ri13/1 -> Reinterpret27/1
  Gateway Out45/1 -> To Workspace45/1
  Reinterpret28/1 -> Gateway Out45/1
  Gateway Out46/1 -> To Workspace46/1
  Reinterpret29/1 -> Gateway Out46/1
  c_to_ri15/2 -> Reinterpret28/1
  c_to_ri15/1 -> Reinterpret29/1
  bus_expand5/2(out3) -> c_to_ri13/1
  bus_expand5/3(out2) -> c_to_ri15/1
  Gateway Out47/1 -> To Workspace47/1
  Reinterpret30/1 -> Gateway Out47/1
  Gateway Out48/1 -> To Workspace48/1
  Reinterpret31/1 -> Gateway Out48/1
  c_to_ri16/2 -> Reinterpret30/1
  c_to_ri16/1 -> Reinterpret31/1
  bus_expand5/4(lsb_out1) -> c_to_ri16/1
  Gateway Out50/1 -> To Workspace49/1
  Reinterpret48/1 -> Gateway Out50/1
  Gateway Out51/1 -> To Workspace51/1
  Reinterpret41/1 -> Gateway Out51/1
  c_to_ri22/2 -> Reinterpret48/1
  c_to_ri22/1 -> Reinterpret41/1
  bus_expand6/1(msb_out4) -> c_to_ri22/1
  Gateway Out49/1 -> To Workspace50/1
  Reinterpret42/1 -> Gateway Out49/1
  Gateway Out52/1 -> To Workspace52/1
  Reinterpret43/1 -> Gateway Out52/1
  c_to_ri21/2 -> Reinterpret42/1
  c_to_ri21/1 -> Reinterpret43/1
  Gateway Out53/1 -> To Workspace53/1
  Reinterpret44/1 -> Gateway Out53/1
  Gateway Out54/1 -> To Workspace54/1
  Reinterpret45/1 -> Gateway Out54/1
  c_to_ri23/2 -> Reinterpret44/1
  c_to_ri23/1 -> Reinterpret45/1
  bus_expand6/2(out3) -> c_to_ri21/1
  bus_expand6/3(out2) -> c_to_ri23/1
  Gateway Out55/1 -> To Workspace55/1
  Reinterpret46/1 -> Gateway Out55/1
  Gateway Out56/1 -> To Workspace56/1
  Reinterpret47/1 -> Gateway Out56/1
  c_to_ri24/2 -> Reinterpret46/1
  c_to_ri24/1 -> Reinterpret47/1
  bus_expand6/4(lsb_out1) -> c_to_ri24/1
  Gateway Out58/1 -> To Workspace57/1
  Reinterpret56/1 -> Gateway Out58/1
  Gateway Out59/1 -> To Workspace59/1
  Reinterpret49/1 -> Gateway Out59/1
  c_to_ri26/2 -> Reinterpret56/1
  c_to_ri26/1 -> Reinterpret49/1
  bus_expand7/1(msb_out4) -> c_to_ri26/1
  Gateway Out57/1 -> To Workspace58/1
  Reinterpret50/1 -> Gateway Out57/1
  Gateway Out60/1 -> To Workspace60/1
  Reinterpret51/1 -> Gateway Out60/1
  c_to_ri25/2 -> Reinterpret50/1
  c_to_ri25/1 -> Reinterpret51/1
  Gateway Out61/1 -> To Workspace61/1
  Reinterpret52/1 -> Gateway Out61/1
  Gateway Out62/1 -> To Workspace62/1
  Reinterpret53/1 -> Gateway Out62/1
  c_to_ri27/2 -> Reinterpret52/1
  c_to_ri27/1 -> Reinterpret53/1
  bus_expand7/2(out3) -> c_to_ri25/1
  bus_expand7/3(out2) -> c_to_ri27/1
  Gateway Out63/1 -> To Workspace63/1
  Reinterpret54/1 -> Gateway Out63/1
  Gateway Out64/1 -> To Workspace64/1
  Reinterpret55/1 -> Gateway Out64/1
  c_to_ri28/2 -> Reinterpret54/1
  c_to_ri28/1 -> Reinterpret55/1
  bus_expand7/4(lsb_out1) -> c_to_ri28/1
  Gateway Out66/1 -> To Workspace65/1
  Reinterpret64/1 -> Gateway Out66/1
  Gateway Out67/1 -> To Workspace67/1
  Reinterpret57/1 -> Gateway Out67/1
  c_to_ri30/2 -> Reinterpret64/1
  c_to_ri30/1 -> Reinterpret57/1
  bus_expand8/1(msb_out4) -> c_to_ri30/1
  Gateway Out65/1 -> To Workspace66/1
  Reinterpret58/1 -> Gateway Out65/1
  Gateway Out68/1 -> To Workspace68/1
  Reinterpret59/1 -> Gateway Out68/1
  c_to_ri29/2 -> Reinterpret58/1
  c_to_ri29/1 -> Reinterpret59/1
  Gateway Out69/1 -> To Workspace69/1
  Reinterpret60/1 -> Gateway Out69/1
  Gateway Out70/1 -> To Workspace70/1
  Reinterpret61/1 -> Gateway Out70/1
  c_to_ri31/2 -> Reinterpret60/1
  c_to_ri31/1 -> Reinterpret61/1
  bus_expand8/2(out3) -> c_to_ri29/1
  bus_expand8/3(out2) -> c_to_ri31/1
  Gateway Out71/1 -> To Workspace71/1
  Reinterpret62/1 -> Gateway Out71/1
  Gateway Out72/1 -> To Workspace72/1
  Reinterpret63/1 -> Gateway Out72/1
  c_to_ri32/2 -> Reinterpret62/1
  c_to_ri32/1 -> Reinterpret63/1
  bus_expand8/4(lsb_out1) -> c_to_ri32/1
  pipeline11/1(q) -> hilbert0/1(a)
  pipeline1/1(q) -> hilbert0/2(b)
  pipeline2/1(q) -> hilbert1/1(a)
  pipeline3/1(q) -> hilbert1/2(b)
  pipeline4/1(q) -> hilbert1/3(misci), hilbert0/3(misci)
